simulink model "PrkgClimaActvMgr"  (R2022b) (continued)
== system PrkgClimaActvMgr == (continued)
  LDACState_LDACState/1 -> PrkgClimaActvMgr_Runnable_sys/153(LDACState_LDACState_read)
  MemBookTrvlClimaTi_MemBookTrvlClimaTi/1 -> PrkgClimaActvMgr_Runnable_sys/154(MemBookTrvlClimaTi_MemBookTrvlClimaTi_read)
  MemReadForBlwAftRun_MemReadForBlwAftRun/1 -> PrkgClimaActvMgr_Runnable_sys/155(MemReadForBlwAftRun_MemReadForBlwAftRun_read)
  MemReadForHighTempCln_MemReadForHighTempCln/1 -> PrkgClimaActvMgr_Runnable_sys/156(MemReadForHighTempCln_MemReadForHighTempCln_read)
  MemReadForHMISetEngReq_MemReadForHMISetEngReq/1 -> PrkgClimaActvMgr_Runnable_sys/157(MemReadForHMISetEngReq_MemReadForHMISetEngReq_read)
  MemReadForParkVent_MemReadForParkVent/1 -> PrkgClimaActvMgr_Runnable_sys/158(MemReadForParkVent_MemReadForParkVent_read)
  MemReadForParkVentActvCnt_MemReadForParkVentActvCnt/1 -> PrkgClimaActvMgr_Runnable_sys/159(MemReadForParkVentActvCnt_MemReadForParkVentActvCnt_read)
  OnBdChrgrSt_OnBdChrgrSt/1 -> PrkgClimaActvMgr_Runnable_sys/160(OnBdChrgrSt_OnBdChrgrSt_read)
  OnBdChrgrSt_OnBdChrgrSt_IsUpdated/1 -> PrkgClimaActvMgr_Runnable_sys/161(OnBdChrgrSt_OnBdChrgrSt_IsUpdated_read)
  OxygenSplyAvlStsNgcc_OxygenSplyAvlStsNgcc/1 -> PrkgClimaActvMgr_Runnable_sys/162(OxygenSplyAvlStsNgcc_OxygenSplyAvlStsNgcc_read)
  OxygenSplyErrNgcc_OxygenSplyErrNgcc/1 -> PrkgClimaActvMgr_Runnable_sys/163(OxygenSplyErrNgcc_OxygenSplyErrNgcc_read)
  PassSeatHeatgLvlSts_PassSeatHeatgLvlSts/1 -> PrkgClimaActvMgr_Runnable_sys/164(PassSeatHeatgLvlSts_PassSeatHeatgLvlSts_read)
  PassSeatHeatgLvlSts_PassSeatHeatgLvlSts_IsUpdated/1 -> PrkgClimaActvMgr_Runnable_sys/165(PassSeatHeatgLvlSts_PassSeatHeatgLvlSts_IsUpdated_read)
  PassSeatVentnLvlSts_PassSeatVentnLvlSts/1 -> PrkgClimaActvMgr_Runnable_sys/166(PassSeatVentnLvlSts_PassSeatVentnLvlSts_read)
  PassSeatVentnLvlSts_PassSeatVentnLvlSts_IsUpdated/1 -> PrkgClimaActvMgr_Runnable_sys/167(PassSeatVentnLvlSts_PassSeatVentnLvlSts_IsUpdated_read)
  PassSeatVentnLvlSts2Ngcc_PassSeatVentnLvlSts2Ngcc/1 -> PrkgClimaActvMgr_Runnable_sys/168(PassSeatVentnLvlSts2Ngcc_PassSeatVentnLvlSts2Ngcc_read)
  PrkgClimaReqExcpSeat_PrkgClimaReqExcpSeat/1 -> PrkgClimaActvMgr_Runnable_sys/169(PrkgClimaReqExcpSeat_PrkgClimaReqExcpSeat_read)
  PrkgClimaReqPrio_PrkgClimaReqPrio/1 -> PrkgClimaActvMgr_Runnable_sys/170(PrkgClimaReqPrio_PrkgClimaReqPrio_read)
  PrkgOGMAirSrcReq_PrkgOGMAirSrcReq/1 -> PrkgClimaActvMgr_Runnable_sys/171(PrkgOGMAirSrcReq_PrkgOGMAirSrcReq_read)
  PrvntWinActvReq_PrvntWinActvReq/1 -> PrkgClimaActvMgr_Runnable_sys/172(PrvntWinActvReq_PrvntWinActvReq_read)
  ReHvacBlowerSts_ReHvacBlowerSts/1 -> PrkgClimaActvMgr_Runnable_sys/173(ReHvacBlowerSts_ReHvacBlowerSts_read)
  RemClimaEgyExsrcReqPlus_RemClimaEgyExsrcReqPlus/1 -> PrkgClimaActvMgr_Runnable_sys/174(RemClimaEgyExsrcReqPlus_RemClimaEgyExsrcReqPlus_read)
  RemClimaHVReqFb_RemClimaHVReqFb/1 -> PrkgClimaActvMgr_Runnable_sys/175(RemClimaHVReqFb_RemClimaHVReqFb_read)
  RemClimaHVReqFb_RemClimaHVReqFb_IsUpdated/1 -> PrkgClimaActvMgr_Runnable_sys/176(RemClimaHVReqFb_RemClimaHVReqFb_IsUpdated_read)
  RemClimaWarnForCmptmtClng_RemClimaWarnForCmptmtClng/1 -> PrkgClimaActvMgr_Runnable_sys/177(RemClimaWarnForCmptmtClng_RemClimaWarnForCmptmtClng_read)
  RemClimaWarnForOpenGlb_RemClimaWarnForOpenGlb/1 -> PrkgClimaActvMgr_Runnable_sys/178(RemClimaWarnForOpenGlb_RemClimaWarnForOpenGlb_read)
  RemClimaWarnForUnLckClima_RemClimaWarnForUnLckClima/1 -> PrkgClimaActvMgr_Runnable_sys/179(RemClimaWarnForUnLckClima_RemClimaWarnForUnLckClima_read)
  RemOxygenSplyReq_RemOxygenSplyReq/1 -> PrkgClimaActvMgr_Runnable_sys/180(RemOxygenSplyReq_RemOxygenSplyReq_read)
  SeatHeatgLvlStsRowSecLe_SeatHeatgLvlStsRowSecLe/1 -> PrkgClimaActvMgr_Runnable_sys/181(SeatHeatgLvlStsRowSecLe_SeatHeatgLvlStsRowSecLe_read)
  SeatHeatgLvlStsRowSecLe_SeatHeatgLvlStsRowSecLe_IsUpdated/1 -> PrkgClimaActvMgr_Runnable_sys/182(SeatHeatgLvlStsRowSecLe_SeatHeatgLvlStsRowSecLe_IsUpdated_read)
  SeatHeatgLvlStsRowSecRi_SeatHeatgLvlStsRowSecRi/1 -> PrkgClimaActvMgr_Runnable_sys/183(SeatHeatgLvlStsRowSecRi_SeatHeatgLvlStsRowSecRi_read)
  SeatHeatgLvlStsRowSecRi_SeatHeatgLvlStsRowSecRi_IsUpdated/1 -> PrkgClimaActvMgr_Runnable_sys/184(SeatHeatgLvlStsRowSecRi_SeatHeatgLvlStsRowSecRi_IsUpdated_read)
  SeatHeatgLvlStsRowThrdLe_SeatHeatgLvlStsRowThrdLe/1 -> PrkgClimaActvMgr_Runnable_sys/185(SeatHeatgLvlStsRowThrdLe_SeatHeatgLvlStsRowThrdLe_read)
  SeatHeatgLvlStsRowThrdLe_SeatHeatgLvlStsRowThrdLe_IsUpdated/1 -> PrkgClimaActvMgr_Runnable_sys/186(SeatHeatgLvlStsRowThrdLe_SeatHeatgLvlStsRowThrdLe_IsUpdated_read)
  SeatHeatgLvlStsRowThrdRi_SeatHeatgLvlStsRowThrdRi/1 -> PrkgClimaActvMgr_Runnable_sys/187(SeatHeatgLvlStsRowThrdRi_SeatHeatgLvlStsRowThrdRi_read)
  SeatHeatgLvlStsRowThrdRi_SeatHeatgLvlStsRowThrdRi_IsUpdated/1 -> PrkgClimaActvMgr_Runnable_sys/188(SeatHeatgLvlStsRowThrdRi_SeatHeatgLvlStsRowThrdRi_IsUpdated_read)
  SeatVentnLvlStsRowSecLe_SeatVentnLvlStsRowSecLe/1 -> PrkgClimaActvMgr_Runnable_sys/189(SeatVentnLvlStsRowSecLe_SeatVentnLvlStsRowSecLe_read)
  SeatVentnLvlStsRowSecLe_SeatVentnLvlStsRowSecLe_IsUpdated/1 -> PrkgClimaActvMgr_Runnable_sys/190(SeatVentnLvlStsRowSecLe_SeatVentnLvlStsRowSecLe_IsUpdated_read)
  SeatVentnLvlStsRowSecRi_SeatVentnLvlStsRowSecRi/1 -> PrkgClimaActvMgr_Runnable_sys/191(SeatVentnLvlStsRowSecRi_SeatVentnLvlStsRowSecRi_read)
  SeatVentnLvlStsRowSecRi_SeatVentnLvlStsRowSecRi_IsUpdated/1 -> PrkgClimaActvMgr_Runnable_sys/192(SeatVentnLvlStsRowSecRi_SeatVentnLvlStsRowSecRi_IsUpdated_read)
  SeatVentnLvlStsRowThrdLe_SeatVentnLvlStsRowThrdLe/1 -> PrkgClimaActvMgr_Runnable_sys/193(SeatVentnLvlStsRowThrdLe_SeatVentnLvlStsRowThrdLe_read)
  SeatVentnLvlStsRowThrdLe_SeatVentnLvlStsRowThrdLe_IsUpdated/1 -> PrkgClimaActvMgr_Runnable_sys/194(SeatVentnLvlStsRowThrdLe_SeatVentnLvlStsRowThrdLe_IsUpdated_read)
  SeatVentnLvlStsRowThrdRi_SeatVentnLvlStsRowThrdRi/1 -> PrkgClimaActvMgr_Runnable_sys/195(SeatVentnLvlStsRowThrdRi_SeatVentnLvlStsRowThrdRi_read)
  SeatVentnLvlStsRowThrdRi_SeatVentnLvlStsRowThrdRi_IsUpdated/1 -> PrkgClimaActvMgr_Runnable_sys/196(SeatVentnLvlStsRowThrdRi_SeatVentnLvlStsRowThrdRi_IsUpdated_read)
  SteerWhlHeatgDurgClimaEnadFromExsrc_SteerWhlHeatgDurgClimaEnadFromExsrc/1 -> PrkgClimaActvMgr_Runnable_sys/197(SteerWhlHeatgDurgClimaEnadFromExsrc_SteerWhlHeatgDurgClimaEnadFromExsrc_read)
  SunRoofPosnSts_Ngcc_SunRoofPosnSts/1 -> PrkgClimaActvMgr_Runnable_sys/198(SunRoofPosnSts_Ngcc_SunRoofPosnSts_read)
  TcsOvrheatdProtnReq_TcsOvrheatdProtnReq/1 -> PrkgClimaActvMgr_Runnable_sys/199(TcsOvrheatdProtnReq_TcsOvrheatdProtnReq_read)
  TelmClimaTmrFromExtSrc_TelmClimaTmrFromExtSrc/1 -> PrkgClimaActvMgr_Runnable_sys/200(TelmClimaTmrFromExtSrc_TelmClimaTmrFromExtSrc_read)
  TelmDefrostTmr_TelmDefrostTmr/1 -> PrkgClimaActvMgr_Runnable_sys/201(TelmDefrostTmr_TelmDefrostTmr_read)
  TelmDefrostTmr_TelmDefrostTmr_IsUpdated/1 -> PrkgClimaActvMgr_Runnable_sys/202(TelmDefrostTmr_TelmDefrostTmr_IsUpdated_read)
  TelmOxygenReqFromExSrc_TelmOxygenReqFromExSrc/1 -> PrkgClimaActvMgr_Runnable_sys/203(TelmOxygenReqFromExSrc_TelmOxygenReqFromExSrc_read)
  TelmOxygenTmr_TelmOxygenTmr/1 -> PrkgClimaActvMgr_Runnable_sys/204(TelmOxygenTmr_TelmOxygenTmr_read)
  TelmOxygenTmr_TelmOxygenTmr_IsUpdated/1 -> PrkgClimaActvMgr_Runnable_sys/205(TelmOxygenTmr_TelmOxygenTmr_IsUpdated_read)
  TelmSeatDrvrHeatReqFromExsrc_TelmSeatDrvrHeatReqFromExsrc/1 -> PrkgClimaActvMgr_Runnable_sys/206(TelmSeatDrvrHeatReqFromExsrc_TelmSeatDrvrHeatReqFromExsrc_read)
  TelmSeatDrvVentnReqFromExsrc_TelmSeatDrvVentnReqFromExsrc/1 -> PrkgClimaActvMgr_Runnable_sys/207(TelmSeatDrvVentnReqFromExsrc_TelmSeatDrvVentnReqFromExsrc_read)
  TelmSeatHeatDrvrTmr_TelmSeatHeatDrvrTmr/1 -> PrkgClimaActvMgr_Runnable_sys/208(TelmSeatHeatDrvrTmr_TelmSeatHeatDrvrTmr_read)
  TelmSeatHeatDrvrTmr_TelmSeatHeatDrvrTmr_IsUpdated/1 -> PrkgClimaActvMgr_Runnable_sys/209(TelmSeatHeatDrvrTmr_TelmSeatHeatDrvrTmr_IsUpdated_read)
  TelmSeatHeatPassTmr_TelmSeatHeatPassTmr/1 -> PrkgClimaActvMgr_Runnable_sys/210(TelmSeatHeatPassTmr_TelmSeatHeatPassTmr_read)
  TelmSeatHeatPassTmr_TelmSeatHeatPassTmr_IsUpdated/1 -> PrkgClimaActvMgr_Runnable_sys/211(TelmSeatHeatPassTmr_TelmSeatHeatPassTmr_IsUpdated_read)
  TelmSeatHeatSecLeTmr_TelmSeatHeatSecLeTmr/1 -> PrkgClimaActvMgr_Runnable_sys/212(TelmSeatHeatSecLeTmr_TelmSeatHeatSecLeTmr_read)
  TelmSeatHeatSecLeTmr_TelmSeatHeatSecLeTmr_IsUpdated/1 -> PrkgClimaActvMgr_Runnable_sys/213(TelmSeatHeatSecLeTmr_TelmSeatHeatSecLeTmr_IsUpdated_read)
  TelmSeatHeatSecRiTmr_TelmSeatHeatSecRiTmr/1 -> PrkgClimaActvMgr_Runnable_sys/214(TelmSeatHeatSecRiTmr_TelmSeatHeatSecRiTmr_read)
  TelmSeatHeatSecRiTmr_TelmSeatHeatSecRiTmr_IsUpdated/1 -> PrkgClimaActvMgr_Runnable_sys/215(TelmSeatHeatSecRiTmr_TelmSeatHeatSecRiTmr_IsUpdated_read)
  TelmSeatHeatThrdLeTmr_TelmSeatHeatThrdLeTmr/1 -> PrkgClimaActvMgr_Runnable_sys/216(TelmSeatHeatThrdLeTmr_TelmSeatHeatThrdLeTmr_read)
  TelmSeatHeatThrdLeTmr_TelmSeatHeatThrdLeTmr_IsUpdated/1 -> PrkgClimaActvMgr_Runnable_sys/217(TelmSeatHeatThrdLeTmr_TelmSeatHeatThrdLeTmr_IsUpdated_read)
  TelmSeatHeatThrdRiTmr_TelmSeatHeatThrdRiTmr/1 -> PrkgClimaActvMgr_Runnable_sys/218(TelmSeatHeatThrdRiTmr_TelmSeatHeatThrdRiTmr_read)
  TelmSeatHeatThrdRiTmr_TelmSeatHeatThrdRiTmr_IsUpdated/1 -> PrkgClimaActvMgr_Runnable_sys/219(TelmSeatHeatThrdRiTmr_TelmSeatHeatThrdRiTmr_IsUpdated_read)
  TelmSeatPassHeatReqFromExsrc_TelmSeatPassHeatReqFromExsrc/1 -> PrkgClimaActvMgr_Runnable_sys/220(TelmSeatPassHeatReqFromExsrc_TelmSeatPassHeatReqFromExsrc_read)
  TelmSeatPassVentnReqFromExsrc_TelmSeatPassVentnReqFromExsrc/1 -> PrkgClimaActvMgr_Runnable_sys/221(TelmSeatPassVentnReqFromExsrc_TelmSeatPassVentnReqFromExsrc_read)
  TelmSeatSecLeHeatReqFromExsrc_TelmSeatSecLeHeatReqFromExsrc/1 -> PrkgClimaActvMgr_Runnable_sys/222(TelmSeatSecLeHeatReqFromExsrc_TelmSeatSecLeHeatReqFromExsrc_read)
  TelmSeatSecLeVentnReqFromExsrc_TelmSeatSecLeVentnReqFromExsrc/1 -> PrkgClimaActvMgr_Runnable_sys/223(TelmSeatSecLeVentnReqFromExsrc_TelmSeatSecLeVentnReqFromExsrc_read)
  TelmSeatSecRiHeatReqFromExsrc_TelmSeatSecRiHeatReqFromExsrc/1 -> PrkgClimaActvMgr_Runnable_sys/224(TelmSeatSecRiHeatReqFromExsrc_TelmSeatSecRiHeatReqFromExsrc_read)
  TelmSeatSecRiVentnReqFromExsrc_TelmSeatSecRiVentnReqFromExsrc/1 -> PrkgClimaActvMgr_Runnable_sys/225(TelmSeatSecRiVentnReqFromExsrc_TelmSeatSecRiVentnReqFromExsrc_read)
  TelmSeatThrdLeHeatReqFromExsrc_TelmSeatThrdLeHeatReqFromExsrc/1 -> PrkgClimaActvMgr_Runnable_sys/226(TelmSeatThrdLeHeatReqFromExsrc_TelmSeatThrdLeHeatReqFromExsrc_read)
  TelmSeatThrdLeVentnReqFromExsrc_TelmSeatThrdLeVentnReqFromExsrc/1 -> PrkgClimaActvMgr_Runnable_sys/227(TelmSeatThrdLeVentnReqFromExsrc_TelmSeatThrdLeVentnReqFromExsrc_read)
  TelmSeatThrdRiHeatReqFromExsrc_TelmSeatThrdRiHeatReqFromExsrc/1 -> PrkgClimaActvMgr_Runnable_sys/228(TelmSeatThrdRiHeatReqFromExsrc_TelmSeatThrdRiHeatReqFromExsrc_read)
  TelmSeatThrdRiVentnReqFromExsrc_TelmSeatThrdRiVentnReqFromExsrc/1 -> PrkgClimaActvMgr_Runnable_sys/229(TelmSeatThrdRiVentnReqFromExsrc_TelmSeatThrdRiVentnReqFromExsrc_read)
  TelmSeatVentnDrvrTmr_TelmSeatVentnDrvrTmr/1 -> PrkgClimaActvMgr_Runnable_sys/230(TelmSeatVentnDrvrTmr_TelmSeatVentnDrvrTmr_read)
  TelmSeatVentnDrvrTmr_TelmSeatVentnDrvrTmr_IsUpdated/1 -> PrkgClimaActvMgr_Runnable_sys/231(TelmSeatVentnDrvrTmr_TelmSeatVentnDrvrTmr_IsUpdated_read)
  TelmSeatVentnPassTmr_TelmSeatVentnPassTmr/1 -> PrkgClimaActvMgr_Runnable_sys/232(TelmSeatVentnPassTmr_TelmSeatVentnPassTmr_read)
  TelmSeatVentnPassTmr_TelmSeatVentnPassTmr_IsUpdated/1 -> PrkgClimaActvMgr_Runnable_sys/233(TelmSeatVentnPassTmr_TelmSeatVentnPassTmr_IsUpdated_read)
  TelmSeatVentnSecLeTmr_TelmSeatVentnSecLeTmr/1 -> PrkgClimaActvMgr_Runnable_sys/234(TelmSeatVentnSecLeTmr_TelmSeatVentnSecLeTmr_read)
  TelmSeatVentnSecLeTmr_TelmSeatVentnSecLeTmr_IsUpdated/1 -> PrkgClimaActvMgr_Runnable_sys/235(TelmSeatVentnSecLeTmr_TelmSeatVentnSecLeTmr_IsUpdated_read)
  TelmSeatVentnSecRiTmr_TelmSeatVentnSecRiTmr/1 -> PrkgClimaActvMgr_Runnable_sys/236(TelmSeatVentnSecRiTmr_TelmSeatVentnSecRiTmr_read)
  TelmSeatVentnSecRiTmr_TelmSeatVentnSecRiTmr_IsUpdated/1 -> PrkgClimaActvMgr_Runnable_sys/237(TelmSeatVentnSecRiTmr_TelmSeatVentnSecRiTmr_IsUpdated_read)
  TelmSeatVentnThrdLeTmr_TelmSeatVentnThrdLeTmr/1 -> PrkgClimaActvMgr_Runnable_sys/238(TelmSeatVentnThrdLeTmr_TelmSeatVentnThrdLeTmr_read)
  TelmSeatVentnThrdLeTmr_TelmSeatVentnThrdLeTmr_IsUpdated/1 -> PrkgClimaActvMgr_Runnable_sys/239(TelmSeatVentnThrdLeTmr_TelmSeatVentnThrdLeTmr_IsUpdated_read)
  TelmSeatVentnThrdReq_TelmSeatVentnThrdReq/1 -> PrkgClimaActvMgr_Runnable_sys/240(TelmSeatVentnThrdReq_TelmSeatVentnThrdReq_read)
  TelmSeatVentnThrdRiTmr_TelmSeatVentnThrdRiTmr/1 -> PrkgClimaActvMgr_Runnable_sys/241(TelmSeatVentnThrdRiTmr_TelmSeatVentnThrdRiTmr_read)
  TelmSeatVentnThrdRiTmr_TelmSeatVentnThrdRiTmr_IsUpdated/1 -> PrkgClimaActvMgr_Runnable_sys/242(TelmSeatVentnThrdRiTmr_TelmSeatVentnThrdRiTmr_IsUpdated_read)
  TelmSteerWhlHeatTmr_TelmSteerWhlHeatTmr/1 -> PrkgClimaActvMgr_Runnable_sys/243(TelmSteerWhlHeatTmr_TelmSteerWhlHeatTmr_read)
  TelmSteerWhlHeatTmr_TelmSteerWhlHeatTmr_IsUpdated/1 -> PrkgClimaActvMgr_Runnable_sys/244(TelmSteerWhlHeatTmr_TelmSteerWhlHeatTmr_IsUpdated_read)
  UnLckClimaActvReq_UnLckClimaActvReq/1 -> PrkgClimaActvMgr_Runnable_sys/245(UnLckClimaActvReq_UnLckClimaActvReq_read)
  UnLckClimaActvReqSt_UnLckClimaActvReqSt/1 -> PrkgClimaActvMgr_Runnable_sys/246(UnLckClimaActvReqSt_UnLckClimaActvReqSt_read)
  UnLckHeatActvReq_UnLckHeatActvReq/1 -> PrkgClimaActvMgr_Runnable_sys/247(UnLckHeatActvReq_UnLckHeatActvReq_read)
  UnLckHeatActvReqSt_UnLckHeatActvReqSt/1 -> PrkgClimaActvMgr_Runnable_sys/248(UnLckHeatActvReqSt_UnLckHeatActvReqSt_read)
  VentnForWinOpenGlbActv_VentnForWinOpenGlbActv/1 -> PrkgClimaActvMgr_Runnable_sys/249(VentnForWinOpenGlbActv_VentnForWinOpenGlbActv_read)
  VFCPrkDrvgClimabyOxygenSplyCtrl_VFCPrkDrvgClimabyOxygenSplyCtrl/1 -> PrkgClimaActvMgr_Runnable_sys/250(VFCPrkDrvgClimabyOxygenSplyCtrl_VFCPrkDrvgClimabyOxygenSplyCtrl_read)
  WarnLvlMsgNgcc_WarnLvlMsgNgcc/1 -> PrkgClimaActvMgr_Runnable_sys/251(WarnLvlMsgNgcc_WarnLvlMsgNgcc_read)
  WarnLvlMsgNgcc_WarnLvlMsgNgcc_IsUpdated/1 -> PrkgClimaActvMgr_Runnable_sys/252(WarnLvlMsgNgcc_WarnLvlMsgNgcc_IsUpdated_read)
  WinOpenGlbReq_WinOpenGlbReq/1 -> PrkgClimaActvMgr_Runnable_sys/253(WinOpenGlbReq_WinOpenGlbReq_read)
  WinPosnStsAtReLe_WinPosnStsAtReLe/1 -> PrkgClimaActvMgr_Runnable_sys/254(WinPosnStsAtReLe_WinPosnStsAtReLe_read)
  WinPosnStsAtReRi_WinPosnStsAtReRi/1 -> PrkgClimaActvMgr_Runnable_sys/255(WinPosnStsAtReRi_WinPosnStsAtReRi_read)
  WinPosnStsDrv_WinPosnStsAtDrv/1 -> PrkgClimaActvMgr_Runnable_sys/256(WinPosnStsDrv_WinPosnStsAtDrv_read)
  WinPosnStsPass_WinPosnStsAtPass/1 -> PrkgClimaActvMgr_Runnable_sys/257(WinPosnStsPass_WinPosnStsAtPass_read)
  PrkgClimaActvMgr_Runnable_sys/1(BlwAftRunAct_BlwAftRunAct_write) -> BlwAftRunAct_BlwAftRunAct/1
  PrkgClimaActvMgr_Runnable_sys/2(BlwAftRunReqRe_BlwAftRunReqRe_write) -> BlwAftRunReqRe_BlwAftRunReqRe/1
  PrkgClimaActvMgr_Runnable_sys/3(BlwAftRunWkTime_BlwAftRunWkTime_write) -> BlwAftRunWkTime_BlwAftRunWkTime/1
  PrkgClimaActvMgr_Runnable_sys/4(BookTrvlClimaFnshd_BookTrvlClimaFnshd_write) -> BookTrvlClimaFnshd_BookTrvlClimaFnshd/1
  PrkgClimaActvMgr_Runnable_sys/5(DsChrgClima_DsChrgClima_write) -> DsChrgClima_DsChrgClima/1
  PrkgClimaActvMgr_Runnable_sys/6(HghClnAct_HghClnAct_write) -> HghClnAct_HghClnAct/1
  PrkgClimaActvMgr_Runnable_sys/7(HghClnWkTime_HghClnWkTime_write) -> HghClnWkTime_HghClnWkTime/1
  PrkgClimaActvMgr_Runnable_sys/8(HighTempCleanRunningStsNgcc_HighTempCleanRunningStsNgcc_write) -> HighTempCleanRunningStsNgcc_HighTempCleanRunningStsNgcc/1
  PrkgClimaActvMgr_Runnable_sys/9(HighTempCleanSettingStsNgcc_HighTempCleanSettingStsNgcc_write) -> HighTempCleanSettingStsNgcc_HighTempCleanSettingStsNgcc/1
  PrkgClimaActvMgr_Runnable_sys/10(HighTempClnActv_HighTempClnActv_write) -> HighTempClnActv_HighTempClnActv/1
  PrkgClimaActvMgr_Runnable_sys/11(KeyClimaActvFnshd_KeyClimaActvFnshd_write) -> KeyClimaActvFnshd_KeyClimaActvFnshd/1
  PrkgClimaActvMgr_Runnable_sys/12(KeySynchrnztnLclNgcc_KeySynchrnztnLclNgcc_write) -> KeySynchrnztnLclNgcc_KeySynchrnztnLclNgcc/1
  PrkgClimaActvMgr_Runnable_sys/13(MemSaveFlgForBlwAftRun_MemSaveFlgForBlwAftRun_write) -> MemSaveFlgForBlwAftRun_MemSaveFlgForBlwAftRun/1
  PrkgClimaActvMgr_Runnable_sys/14(MemSaveFlgForHighTempCln_MemSaveFlgForHighTempCln_write) -> MemSaveFlgForHighTempCln_MemSaveFlgForHighTempCln/1
  PrkgClimaActvMgr_Runnable_sys/15(MemSaveFlgForHMISetEngReq_MemSaveFlgForHMISetEngReq_write) -> MemSaveFlgForHMISetEngReq_MemSaveFlgForHMISetEngReq/1
  PrkgClimaActvMgr_Runnable_sys/16(MemSaveFlgForParkVent_MemSaveFlgForParkVent_write) -> MemSaveFlgForParkVent_MemSaveFlgForParkVent/1
  PrkgClimaActvMgr_Runnable_sys/17(MemSaveFlgForParkVentActvCnt_MemSaveFlgForParkVentActvCnt_write) -> MemSaveFlgForParkVentActvCnt_MemSaveFlgForParkVentActvCnt/1
  PrkgClimaActvMgr_Runnable_sys/18(MemWrForBlwAftRun_MemWrForBlwAftRun_write) -> MemWrForBlwAftRun_MemWrForBlwAftRun/1
  PrkgClimaActvMgr_Runnable_sys/19(MemWrForHighTempCln_MemWrForHighTempCln_write) -> MemWrForHighTempCln_MemWrForHighTempCln/1
  PrkgClimaActvMgr_Runnable_sys/20(MemWrForHMISetEngReq_MemWrForHMISetEngReq_write) -> MemWrForHMISetEngReq_MemWrForHMISetEngReq/1
  PrkgClimaActvMgr_Runnable_sys/21(MemWrForParkVent_MemWrForParkVent_write) -> MemWrForParkVent_MemWrForParkVent/1
  PrkgClimaActvMgr_Runnable_sys/22(MemWrForParkVentActvCnt_MemWrForParkVentActvCnt_write) -> MemWrForParkVentActvCnt_MemWrForParkVentActvCnt/1
  PrkgClimaActvMgr_Runnable_sys/23(OGMAirSrcFnshd_OGMAirSrcFnshd_write) -> OGMAirSrcFnshd_OGMAirSrcFnshd/1
  PrkgClimaActvMgr_Runnable_sys/24(OGMAirSrcReq_OGMAirSrcReq_write) -> OGMAirSrcReq_OGMAirSrcReq/1
  PrkgClimaActvMgr_Runnable_sys/25(OGMAvlSts_OGMAvlSts_write) -> OGMAvlSts_OGMAvlSts/1
  PrkgClimaActvMgr_Runnable_sys/26(OGMReq_OGMReq_write) -> OGMReq_OGMReq/1
  PrkgClimaActvMgr_Runnable_sys/27(ParkVentActv_ParkVentActv_write) -> ParkVentActv_ParkVentActv/1
  PrkgClimaActvMgr_Runnable_sys/28(ParkVentWkTi_ParkVentWkTi_write) -> ParkVentWkTi_ParkVentWkTi/1
  PrkgClimaActvMgr_Runnable_sys/29(PrkgClimaACReq_PrkgClimaACReq_write) -> PrkgClimaACReq_PrkgClimaACReq/1
  PrkgClimaActvMgr_Runnable_sys/30(PrkgClimaActvMgrVFCLDACClimaCtrl_PrkgClimaActvMgrVFCLDACClimaCtrl_write) -> PrkgClimaActvMgrVFCLDACClimaCtrl_PrkgClimaActvMgrVFCLDACClimaCtrl/1
  PrkgClimaActvMgr_Runnable_sys/31(PrkgClimaAirflowReqRe_PrkgClimaAirflowReqRe_write) -> PrkgClimaAirflowReqRe_PrkgClimaAirflowReqRe/1
  PrkgClimaActvMgr_Runnable_sys/32(PrkgClimaEngStReqNgcc_PrkgClimaEngStReqNgcc_write) -> PrkgClimaEngStReqNgcc_PrkgClimaEngStReqNgcc/1
  PrkgClimaActvMgr_Runnable_sys/33(PrkgClimaHVReq_PrkgClimaHVReq_write) -> PrkgClimaHVReq_PrkgClimaHVReq/1
  PrkgClimaActvMgr_Runnable_sys/34(PrkgClimaOnReqRe_PrkgClimaOnReqRe_write) -> PrkgClimaOnReqRe_PrkgClimaOnReqRe/1
  PrkgClimaActvMgr_Runnable_sys/35(PrkgCmftRlyReq_PrkgCmftRlyReq_write) -> PrkgCmftRlyReq_PrkgCmftRlyReq/1
  PrkgClimaActvMgr_Runnable_sys/36(PrkgOGMAirSrcActv_PrkgOGMAirSrcActv_write) -> PrkgOGMAirSrcActv_PrkgOGMAirSrcActv/1
  PrkgClimaActvMgr_Runnable_sys/37(PrkgVntFctn_PrkgVntFctn_write) -> PrkgVntFctn_PrkgVntFctn/1
  PrkgClimaActvMgr_Runnable_sys/38(PrvntWinFrz_PrvntWinFrz_write) -> PrvntWinFrz_PrvntWinFrz/1
  PrkgClimaActvMgr_Runnable_sys/39(PrvntWinFrzFnshd_PrvntWinFrzFnshd_write) -> PrvntWinFrzFnshd_PrvntWinFrzFnshd/1
  PrkgClimaActvMgr_Runnable_sys/40(RemActvSts_RemActvSts_write) -> RemActvSts_RemActvSts/1
  PrkgClimaActvMgr_Runnable_sys/41(RemClimaFnshd1_RemClimaFnshd1_write) -> RemClimaFnshd1_RemClimaFnshd1/1
  PrkgClimaActvMgr_Runnable_sys/42(RemClimaWarn_RemClimaWarn_write) -> RemClimaWarn_RemClimaWarn/1
  PrkgClimaActvMgr_Runnable_sys/43(RemOxygenSplyActv_RemOxygenSplyActv_write) -> RemOxygenSplyActv_RemOxygenSplyActv/1
  PrkgClimaActvMgr_Runnable_sys/44(RemOxygenSplyActvFaildNgcc_RemOxygenSplyActvFaildNgcc_write) -> RemOxygenSplyActvFaildNgcc_RemOxygenSplyActvFaildNgcc/1
  PrkgClimaActvMgr_Runnable_sys/45(RemOxygenSplyFnshd_RemOxygenSplyFnshd_write) -> RemOxygenSplyFnshd_RemOxygenSplyFnshd/1
  PrkgClimaActvMgr_Runnable_sys/46(RemOxygenSplyFnshd1_RemOxygenSplyFnshd1_write) -> RemOxygenSplyFnshd1_RemOxygenSplyFnshd1/1
  PrkgClimaActvMgr_Runnable_sys/47(RemOxygenSplyWarn_RemOxygenSplyWarn_write) -> RemOxygenSplyWarn_RemOxygenSplyWarn/1
  PrkgClimaActvMgr_Runnable_sys/48(RemSynchrnztnLcl_RemSynchrnztnLcl_write) -> RemSynchrnztnLcl_RemSynchrnztnLcl/1
  PrkgClimaActvMgr_Runnable_sys/49(RemWinClsReq_RemWinClsReq_write) -> RemWinClsReq_RemWinClsReq/1
  PrkgClimaActvMgr_Runnable_sys/50(SeatClimaDurgClimaHmiSeatHeatgForRowThrdLe_SeatClimaDurgClimaHmiSeatHeatgForRowThrdLe_write) -> SeatClimaDurgClimaHmiSeatHeatgForRowThrdLe_SeatClimaDurgClimaHmiSeatHeatgForRowThrdLe/1
  PrkgClimaActvMgr_Runnable_sys/51(SeatClimaDurgClimaHmiSeatHeatgForRowThrdRi_SeatClimaDurgClimaHmiSeatHeatgForRowThrdRi_write) -> SeatClimaDurgClimaHmiSeatHeatgForRowThrdRi_SeatClimaDurgClimaHmiSeatHeatgForRowThrdRi/1
  PrkgClimaActvMgr_Runnable_sys/52(SeatClimaDurgClimaHmiSeatVentnForRowThrdLe_SeatClimaDurgClimaHmiSeatVentnForRowThrdLe_write) -> SeatClimaDurgClimaHmiSeatVentnForRowThrdLe_SeatClimaDurgClimaHmiSeatVentnForRowThrdLe/1
  PrkgClimaActvMgr_Runnable_sys/53(SeatClimaDurgClimaHmiSeatVentnForRowThrdRi_SeatClimaDurgClimaHmiSeatVentnForRowThrdRi_write) -> SeatClimaDurgClimaHmiSeatVentnForRowThrdRi_SeatClimaDurgClimaHmiSeatVentnForRowThrdRi/1
  PrkgClimaActvMgr_Runnable_sys/54(TelmDefrostFnshd_TelmDefrostFnshd_write) -> TelmDefrostFnshd_TelmDefrostFnshd/1
  PrkgClimaActvMgr_Runnable_sys/55(TelmSeatHeatDrvrFnshd_TelmSeatHeatDrvrFnshd_write) -> TelmSeatHeatDrvrFnshd_TelmSeatHeatDrvrFnshd/1
  PrkgClimaActvMgr_Runnable_sys/56(TelmSeatHeatPassFnshd_TelmSeatHeatPassFnshd_write) -> TelmSeatHeatPassFnshd_TelmSeatHeatPassFnshd/1
  PrkgClimaActvMgr_Runnable_sys/57(TelmSeatHeatSecLeFnshd_TelmSeatHeatSecLeFnshd_write) -> TelmSeatHeatSecLeFnshd_TelmSeatHeatSecLeFnshd/1
  PrkgClimaActvMgr_Runnable_sys/58(TelmSeatHeatSecRiFnshd_TelmSeatHeatSecRiFnshd_write) -> TelmSeatHeatSecRiFnshd_TelmSeatHeatSecRiFnshd/1
  PrkgClimaActvMgr_Runnable_sys/59(TelmSeatHeatThrdLeFnshd_TelmSeatHeatThrdLeFnshd_write) -> TelmSeatHeatThrdLeFnshd_TelmSeatHeatThrdLeFnshd/1
  PrkgClimaActvMgr_Runnable_sys/60(TelmSeatHeatThrdRiFnshd_TelmSeatHeatThrdRiFnshd_write) -> TelmSeatHeatThrdRiFnshd_TelmSeatHeatThrdRiFnshd/1
  PrkgClimaActvMgr_Runnable_sys/61(TelmSeatVentnDrvrFnshd_TelmSeatVentnDrvrFnshd_write) -> TelmSeatVentnDrvrFnshd_TelmSeatVentnDrvrFnshd/1
  PrkgClimaActvMgr_Runnable_sys/62(TelmSeatVentnPassFnshd_TelmSeatVentnPassFnshd_write) -> TelmSeatVentnPassFnshd_TelmSeatVentnPassFnshd/1
  PrkgClimaActvMgr_Runnable_sys/63(TelmSeatVentnSecLeFnshd_TelmSeatVentnSecLeFnshd_write) -> TelmSeatVentnSecLeFnshd_TelmSeatVentnSecLeFnshd/1
  PrkgClimaActvMgr_Runnable_sys/64(TelmSeatVentnSecRiFnshd_TelmSeatVentnSecRiFnshd_write) -> TelmSeatVentnSecRiFnshd_TelmSeatVentnSecRiFnshd/1
  PrkgClimaActvMgr_Runnable_sys/65(TelmSeatVentnThrdLeFnshd_TelmSeatVentnThrdLeFnshd_write) -> TelmSeatVentnThrdLeFnshd_TelmSeatVentnThrdLeFnshd/1
  PrkgClimaActvMgr_Runnable_sys/66(TelmSeatVentnThrdRiFnshd_TelmSeatVentnThrdRiFnshd_write) -> TelmSeatVentnThrdRiFnshd_TelmSeatVentnThrdRiFnshd/1
  PrkgClimaActvMgr_Runnable_sys/67(TelmSteerWhlHeatFnshd_TelmSteerWhlHeatFnshd_write) -> TelmSteerWhlHeatFnshd_TelmSteerWhlHeatFnshd/1
  PrkgClimaActvMgr_Runnable_sys/68(VMMChngNdMtnNgcc_VMMChngNdMtnNgcc_write) -> VMMChngNdMtnNgcc_VMMChngNdMtnNgcc/1
  PrkgClimaActvMgr_Runnable_sys/69(VTMOverTProtection_VTMOverTProtection_write) -> VTMOverTProtection_VTMOverTProtection/1
  PrkgClimaActvMgr_Runnable_sys/70(VTMOverTProtectionFnshd_VTMOverTProtectionFnshd_write) -> VTMOverTProtectionFnshd_VTMOverTProtectionFnshd/1
  PrkgClimaActvMgr_Runnable_sys/71(BlwAftRunReq_BlwAftRunReq_write) -> BlwAftRunReq_BlwAftRunReq/1
  PrkgClimaActvMgr_Runnable_sys/72(BlwAftRunSts_BlwAftRunSts_write) -> BlwAftRunSts_BlwAftRunSts/1
  PrkgClimaActvMgr_Runnable_sys/73(BookTrvlClimaActv_BookTrvlClimaActv_write) -> BookTrvlClimaActv_BookTrvlClimaActv/1
  PrkgClimaActvMgr_Runnable_sys/74(CdsParkgClimaActv_CdsParkgClimaActv_write) -> CdsParkgClimaActv_CdsParkgClimaActv/1
  PrkgClimaActvMgr_Runnable_sys/75(ClimaActv_Ngcc_ClimaActv_write) -> ClimaActv_Ngcc_ClimaActv/1
  PrkgClimaActvMgr_Runnable_sys/76(ClimaOvrHeatProActv_ClimaOvrHeatProActv_write) -> ClimaOvrHeatProActv_ClimaOvrHeatProActv/1
  PrkgClimaActvMgr_Runnable_sys/77(ClimaOvrHeatProActvSts_ClimaOvrHeatProActvSts_write) -> ClimaOvrHeatProActvSts_ClimaOvrHeatProActvSts/1
  PrkgClimaActvMgr_Runnable_sys/78(ClimaOvrHeatProWarn_ClimaOvrHeatProWarn_write) -> ClimaOvrHeatProWarn_ClimaOvrHeatProWarn/1
  PrkgClimaActvMgr_Runnable_sys/79(ClimaOvrHeatPrtSts_ClimaOvrHeatPrtSts_write) -> ClimaOvrHeatPrtSts_ClimaOvrHeatPrtSts/1
  PrkgClimaActvMgr_Runnable_sys/80(ClimaSts_ClimaSts_write) -> ClimaSts_ClimaSts/1
  PrkgClimaActvMgr_Runnable_sys/81(ClimaTiOfEnd_Ngcc_ClimaTiOfEnd_write) -> ClimaTiOfEnd_Ngcc_ClimaTiOfEnd/1
  PrkgClimaActvMgr_Runnable_sys/82(ClimaWarn_ClimaWarn_write) -> ClimaWarn_ClimaWarn/1
  PrkgClimaActvMgr_Runnable_sys/83(ClngActv_ClngActv_write) -> ClngActv_ClngActv/1
  Co2WarnForTelm_Co2WarnForTelm_merge/1 -> Co2WarnForTelm_Co2WarnForTelm/1
  PrkgClimaActvMgr_Runnable_sys/85(COHSts_COHSts_write) -> COHSts_COHSts/1
  PrkgClimaActvMgr_Runnable_sys/86(CoolgFastForPrePrkgClimaActv_CoolgFastForPrePrkgClimaActv_write) -> CoolgFastForPrePrkgClimaActv_CoolgFastForPrePrkgClimaActv/1
  PrkgClimaActvMgr_Runnable_sys/87(CoolgFastUniqueForPrePrkgClimaActv_CoolgFastUniqueForPrePrkgClimaActv_write) -> CoolgFastUniqueForPrePrkgClimaActv_CoolgFastUniqueForPrePrkgClimaActv/1
  PrkgClimaActvMgr_Runnable_sys/88(DefrstReq_DefrstReq_write) -> DefrstReq_DefrstReq/1
  PrkgClimaActvMgr_Runnable_sys/89(DiDblLock_DiDblLock_write) -> DiDblLock_DiDblLock/1
  PrkgClimaActvMgr_Runnable_sys/90(EcuKeepActvFromPrkgClima_EcuKeepActvFromPrkgClima_write) -> EcuKeepActvFromPrkgClima_EcuKeepActvFromPrkgClima/1
  PrkgClimaActvMgr_Runnable_sys/91(ExtVentFanVolStsFrmClima_ExtVentFanVolStsFrmClima_write) -> ExtVentFanVolStsFrmClima_ExtVentFanVolStsFrmClima/1
  PrkgClimaActvMgr_Runnable_sys/92(ExtVentStsFrmClima_ExtVentStsFrmClima_write) -> ExtVentStsFrmClima_ExtVentStsFrmClima/1
  ExtVentTempStsFrmClima_ExtVentTempStsFrmClima_merge/1 -> ExtVentTempStsFrmClima_ExtVentTempStsFrmClima/1
  ExtVentTempStsFrmClimaExtVentTemprSts_ExtVentTempStsFrmClimaExtVentTemprSts_merge/1 -> ExtVentTempStsFrmClimaExtVentTemprSts_ExtVentTempStsFrmClimaExtVentTemprSts/1
  ExtVentTempStsFrmClimaExtVentTemprVal_ExtVentTempStsFrmClimaExtVentTemprVal_merge/1 -> ExtVentTempStsFrmClimaExtVentTemprVal_ExtVentTempStsFrmClimaExtVentTemprVal/1
  PrkgClimaActvMgr_Runnable_sys/96(ExtVentToastFrmClima_Prkg_ExtVentToastFrmClima_write) -> ExtVentToastFrmClima_Prkg_ExtVentToastFrmClima/1
  PrkgClimaActvMgr_Runnable_sys/97(HeatrElecFrntReqFromPrkgClima_HeatrElecFrntReqFromPrkgClima_write) -> HeatrElecFrntReqFromPrkgClima_HeatrElecFrntReqFromPrkgClima/1
  PrkgClimaActvMgr_Runnable_sys/98(IntCo2ActvSts_IntCo2ActvSts_write) -> IntCo2ActvSts_IntCo2ActvSts/1
  PrkgClimaActvMgr_Runnable_sys/99(KeyClimaActv_KeyClimaActv_write) -> KeyClimaActv_KeyClimaActv/1
  PrkgClimaActvMgr_Runnable_sys/100(MemSaveFlgForPrkgClimaActvMgr_MemSaveFlgForPrkgClimaActvMgr_write) -> MemSaveFlgForPrkgClimaActvMgr_MemSaveFlgForPrkgClimaActvMgr/1
  MemWrForPrkgClimaActvMgr_MemWrForPrkgClimaActvMgr_merge/1 -> MemWrForPrkgClimaActvMgr_MemWrForPrkgClimaActvMgr/1
  PrkgClimaActvMgr_Runnable_sys/102(ModForPrkgClima_ModForPrkgClima_write) -> ModForPrkgClima_ModForPrkgClima/1
  PrkgClimaActvMgr_Runnable_sys/103(PreClngActv_PreClngActv_write) -> PreClngActv_PreClngActv/1
  PrkgClimaActvMgr_Runnable_sys/104(PreClngNotif_PreClngNotif_write) -> PreClngNotif_PreClngNotif/1
  PrkgClimaActvMgr_Runnable_sys/105(PreClngSts_PreClngSts_write) -> PreClngSts_PreClngSts/1
  PrkgClimaActvMgr_Runnable_sys/106(PrkgClimaActvMgrVFCBodyPreClimatisation_PrkgClimaActvMgrVFCBodyPreClimatisation_write) -> PrkgClimaActvMgrVFCBodyPreClimatisation_PrkgClimaActvMgrVFCBodyPreClimatisation/1
  PrkgClimaActvMgr_Runnable_sys/107(PrkgClimaActvMgrVFCGlobalShortDuration_PrkgClimaActvMgrVFCGlobalShortDuration_write) -> PrkgClimaActvMgrVFCGlobalShortDuration_PrkgClimaActvMgrVFCGlobalShortDuration/1
  PrkgClimaActvMgr_Runnable_sys/108(PrkgClimaActvMgrVFCLocking_PrkgClimaActvMgrVFCLocking_write) -> PrkgClimaActvMgrVFCLocking_PrkgClimaActvMgrVFCLocking/1
  PrkgClimaActvMgr_Runnable_sys/109(PrkgClimaActvMgrVFCParkingDrivingClimatization_PrkgClimaActvMgrVFCParkingDrivingClimatization_write) -> PrkgClimaActvMgrVFCParkingDrivingClimatization_PrkgClimaActvMgrVFCParkingDrivingClimatization/1
  PrkgClimaActvMgr_Runnable_sys/110(PrkgClimaActvMgrVFCPostClimatisation_PrkgClimaActvMgrVFCPostClimatisation_write) -> PrkgClimaActvMgrVFCPostClimatisation_PrkgClimaActvMgrVFCPostClimatisation/1
  PrkgClimaActvMgr_Runnable_sys/111(PrkgClimaActvMgrVFCWindows_PrkgClimaActvMgrVFCWindows_write) -> PrkgClimaActvMgrVFCWindows_PrkgClimaActvMgrVFCWindows/1
  PrkgClimaActvMgr_Runnable_sys/112(PrkgClimaAirflowReq_PrkgClimaAirflowReq_write) -> PrkgClimaAirflowReq_PrkgClimaAirflowReq/1
  PrkgClimaActvMgr_Runnable_sys/113(PrkgClimaFnshd_PrkgClimaFnshd_write) -> PrkgClimaFnshd_PrkgClimaFnshd/1
  PrkgClimaActvMgr_Runnable_sys/114(PrkgClimaHeatgReq_PrkgClimaHeatgReq_write) -> PrkgClimaHeatgReq_PrkgClimaHeatgReq/1
  PrkgClimaActvMgr_Runnable_sys/115(PrkgClimaModReq_PrkgClimaModReq_write) -> PrkgClimaModReq_PrkgClimaModReq/1
  PrkgClimaActvMgr_Runnable_sys/116(PrkgClimaOnReq_PrkgClimaOnReq_write) -> PrkgClimaOnReq_PrkgClimaOnReq/1
  PrkgClimaActvMgr_Runnable_sys/117(PrkgClimaPostCdngReq_PrkgClimaPostCdngReq_write) -> PrkgClimaPostCdngReq_PrkgClimaPostCdngReq/1
  PrkgClimaActvMgr_Runnable_sys/118(PrkgClimaPreCdngReq_PrkgClimaPreCdngReq_write) -> PrkgClimaPreCdngReq_PrkgClimaPreCdngReq/1
  PrkgClimaActvMgr_Runnable_sys/119(PrkgClimaRecirReq_PrkgClimaRecirReq_write) -> PrkgClimaRecirReq_PrkgClimaRecirReq/1
  PrkgClimaActvMgr_Runnable_sys/120(PrkgClimaRqrdImdtForBattULo_PrkgClimaRqrdImdtForBattULo_write) -> PrkgClimaRqrdImdtForBattULo_PrkgClimaRqrdImdtForBattULo/1
  PrkgClimaActvMgr_Runnable_sys/121(PrkgClimaRqrdImdtFromActvMgr_PrkgClimaRqrdImdtFromActvMgr_write) -> PrkgClimaRqrdImdtFromActvMgr_PrkgClimaRqrdImdtFromActvMgr/1
  PrkgClimaActvMgr_Runnable_sys/122(PrkgClimaRqrdTmrForBattULo_PrkgClimaRqrdTmrForBattULo_write) -> PrkgClimaRqrdTmrForBattULo_PrkgClimaRqrdTmrForBattULo/1
  PrkgClimaActvMgr_Runnable_sys/123(PrkgClimaTemp_PrkgClimaTemp_write) -> PrkgClimaTemp_PrkgClimaTemp/1
  PrkgClimaActvMgr_Runnable_sys/124(PrkgClimaWarn_PrkgClimaWarn_write) -> PrkgClimaWarn_PrkgClimaWarn/1
  PrkgClimaActvMgr_Runnable_sys/125(PrkgModMgr_PrkgModMgr_write) -> PrkgModMgr_PrkgModMgr/1
  PrkgClimaActvMgr_Runnable_sys/126(RemClimaActv_RemClimaActv_write) -> RemClimaActv_RemClimaActv/1
  PrkgClimaActvMgr_Runnable_sys/127(RemClimaDefrstSts_RemClimaDefrstSts_write) -> RemClimaDefrstSts_RemClimaDefrstSts/1
  PrkgClimaActvMgr_Runnable_sys/128(RemClimaDelaySts_RemClimaDelaySts_write) -> RemClimaDelaySts_RemClimaDelaySts/1
  PrkgClimaActvMgr_Runnable_sys/129(RemClimaEgyFinalReq_RemClimaEgyFinalReq_write) -> RemClimaEgyFinalReq_RemClimaEgyFinalReq/1
  PrkgClimaActvMgr_Runnable_sys/130(RemClimaExtnTiRspn_RemClimaExtnTiRspn_write) -> RemClimaExtnTiRspn_RemClimaExtnTiRspn/1
  PrkgClimaActvMgr_Runnable_sys/131(RemClimaFnshd_RemClimaFnshd_write) -> RemClimaFnshd_RemClimaFnshd/1
  PrkgClimaActvMgr_Runnable_sys/132(RemClimaHvSts_RemClimaHvSts_write) -> RemClimaHvSts_RemClimaHvSts/1
  PrkgClimaActvMgr_Runnable_sys/133(RemStrtClimaRspn_RemStrtClimaRspn_write) -> RemStrtClimaRspn_RemStrtClimaRspn/1
  PrkgClimaActvMgr_Runnable_sys/134(RemVentActvSts_RemVentActvSts_write) -> RemVentActvSts_RemVentActvSts/1
  PrkgClimaActvMgr_Runnable_sys/135(RemVentFbSts_RemVentFbSts_write) -> RemVentFbSts_RemVentFbSts/1
  PrkgClimaActvMgr_Runnable_sys/136(RemVentReqRspnFb_RemVentReqRspnFb_write) -> RemVentReqRspnFb_RemVentReqRspnFb/1
  PrkgClimaActvMgr_Runnable_sys/137(RemVentWarnSts_RemVentWarnSts_write) -> RemVentWarnSts_RemVentWarnSts/1
  PrkgClimaActvMgr_Runnable_sys/138(ReqForPrkgClima_ReqForPrkgClima_write) -> ReqForPrkgClima_ReqForPrkgClima/1
  PrkgClimaActvMgr_Runnable_sys/139(ReqForSwtHeatrTypFromActvMgr_ReqForSwtHeatrTypFromActvMgr_write) -> ReqForSwtHeatrTypFromActvMgr_ReqForSwtHeatrTypFromActvMgr/1
  PrkgClimaActvMgr_Runnable_sys/140(RoofOpnReqFrmClima_RoofOpnReqFrmClima_write) -> RoofOpnReqFrmClima_RoofOpnReqFrmClima/1
  PrkgClimaActvMgr_Runnable_sys/141(RtLeForPrkgClima_RtLeForPrkgClima_write) -> RtLeForPrkgClima_RtLeForPrkgClima/1
  PrkgClimaActvMgr_Runnable_sys/142(SchedCmptHeatCoolFB_SchedCmptHeatCoolFB_write) -> SchedCmptHeatCoolFB_SchedCmptHeatCoolFB/1
  PrkgClimaActvMgr_Runnable_sys/143(SeatClimaDurgClima_SeatClimaDurgClima_write) -> SeatClimaDurgClima_SeatClimaDurgClima/1
  PrkgClimaActvMgr_Runnable_sys/144(SteerWhlHeatgDurgClima_Ngcc_SteerWhlHeatgDurgClima_write) -> SteerWhlHeatgDurgClima_Ngcc_SteerWhlHeatgDurgClima/1
  PrkgClimaActvMgr_Runnable_sys/145(SuspStrtActvForPrkgClima_SuspStrtActvForPrkgClima_write) -> SuspStrtActvForPrkgClima_SuspStrtActvForPrkgClima/1
  PrkgClimaActvMgr_Runnable_sys/146(WarnForHybWoUFromActvMgr_WarnForHybWoUFromActvMgr_write) -> WarnForHybWoUFromActvMgr_WarnForHybWoUFromActvMgr/1
  PrkgClimaActvMgr_Runnable_sys/101(MemWrForPrkgClimaActvMgr_MemWrForPrkgClimaActvMgr_write) -> [MemWrForPrkgClimaActvMgr_MemWrForPrkgClimaActvMgr_merge_Tag]
  [MemWrForPrkgClimaActvMgr_MemWrForPrkgClimaActvMgr_merge_Tag] -> MemWrForPrkgClimaActvMgr_MemWrForPrkgClimaActvMgr_merge/2
  PrkgClimaActvMgr_Init/5(MemWrForPrkgClimaActvMgr_MemWrForPrkgClimaActvMgr_write) -> [MemWrForPrkgClimaActvMgr_MemWrForPrkgClimaActvMgr_merge_Tag1]
  [MemWrForPrkgClimaActvMgr_MemWrForPrkgClimaActvMgr_merge_Tag1] -> MemWrForPrkgClimaActvMgr_MemWrForPrkgClimaActvMgr_merge/1
  PrkgClimaActvMgr_Runnable_sys/95(ExtVentTempStsFrmClimaExtVentTemprVal_ExtVentTempStsFrmClimaExtVentTemprVal_write) -> [ExtVentTempStsFrmClimaExtVentTemprVal_ExtVentT_f0f4de216a9dedc2]
  [ExtVentTempStsFrmClimaExtVentTemprVal_ExtVentT_f0f4de216a9dedc2] -> ExtVentTempStsFrmClimaExtVentTemprVal_ExtVentTempStsFrmClimaExtVentTemprVal_merge/2
  PrkgClimaActvMgr_Init/4(ExtVentTempStsFrmClimaExtVentTemprVal_ExtVentTempStsFrmClimaExtVentTemprVal_write) -> [ExtVentTempStsFrmClimaExtVentTemprVal_ExtVentT_c32e76e466ed9796]
  [ExtVentTempStsFrmClimaExtVentTemprVal_ExtVentT_c32e76e466ed9796] -> ExtVentTempStsFrmClimaExtVentTemprVal_ExtVentTempStsFrmClimaExtVentTemprVal_merge/1
  PrkgClimaActvMgr_Runnable_sys/94(ExtVentTempStsFrmClimaExtVentTemprSts_ExtVentTempStsFrmClimaExtVentTemprSts_write) -> [ExtVentTempStsFrmClimaExtVentTemprSts_ExtVentT_003a09ad4f08eff1]
  [ExtVentTempStsFrmClimaExtVentTemprSts_ExtVentT_003a09ad4f08eff1] -> ExtVentTempStsFrmClimaExtVentTemprSts_ExtVentTempStsFrmClimaExtVentTemprSts_merge/2
  PrkgClimaActvMgr_Init/3(ExtVentTempStsFrmClimaExtVentTemprSts_ExtVentTempStsFrmClimaExtVentTemprSts_write) -> [ExtVentTempStsFrmClimaExtVentTemprSts_ExtVentT_16af2b52c51322f6]
  [ExtVentTempStsFrmClimaExtVentTemprSts_ExtVentT_16af2b52c51322f6] -> ExtVentTempStsFrmClimaExtVentTemprSts_ExtVentTempStsFrmClimaExtVentTemprSts_merge/1
  PrkgClimaActvMgr_Runnable_sys/93(ExtVentTempStsFrmClima_ExtVentTempStsFrmClima_write) -> [ExtVentTempStsFrmClima_ExtVentTempStsFrmClima_merge_Tag]
  [ExtVentTempStsFrmClima_ExtVentTempStsFrmClima_merge_Tag] -> ExtVentTempStsFrmClima_ExtVentTempStsFrmClima_merge/2
  PrkgClimaActvMgr_Init/2(ExtVentTempStsFrmClima_ExtVentTempStsFrmClima_write) -> [ExtVentTempStsFrmClima_ExtVentTempStsFrmClima_merge_Tag1]
  [ExtVentTempStsFrmClima_ExtVentTempStsFrmClima_merge_Tag1] -> ExtVentTempStsFrmClima_ExtVentTempStsFrmClima_merge/1
  PrkgClimaActvMgr_Runnable_sys/84(Co2WarnForTelm_Co2WarnForTelm_write) -> [Co2WarnForTelm_Co2WarnForTelm_merge_Tag]
  [Co2WarnForTelm_Co2WarnForTelm_merge_Tag] -> Co2WarnForTelm_Co2WarnForTelm_merge/2
  PrkgClimaActvMgr_Init/1(Co2WarnForTelm_Co2WarnForTelm_write) -> [Co2WarnForTelm_Co2WarnForTelm_merge_Tag1]
  [Co2WarnForTelm_Co2WarnForTelm_merge_Tag1] -> Co2WarnForTelm_Co2WarnForTelm_merge/1
  PrkgClimaActvMgr_Runnable/1 -> PrkgClimaActvMgr_Runnable_sys/Trigger
